simulink library "TripedLibraryMAX"  (R2021a)
settings: Solver=VariableStepAuto  StartTime=0.0  StopTime=10.0  RelTol=1e-3
undefined symbols (not defined in the file or in its folder): X, Y, Z
libraries: sm_lib x100, nesl_utility x31, $bdroot x27, robotlib x27, simulink x6
(part 5 of 6)

== system TripedLibraryMAX/Full Model/ROS/Subscribers/Subscriber_leg1/Ros-Subscriber-leg-1-left-swing-motor ==
  Bus Selector : BusSelector  OutputSignals=Position
  Constant : Constant  Value=[1]
  Selector : Selector  IndexOptions=Index vector (port)  Indices=[2]  OutputSizes=1
  Subscribe : robotlib/Subscribe  topicSource=Specify your own  topic=/leg_1/swing/left/joint/position_controller/command  messageType=sensor_msgs/JointState  SampleTime=-1  legacyBusStruct=off  messageQueueLength=1
  Terminator1 : Terminator
  swing_left_motor_position_OUT : Outport
  wires:
  Subscribe/1 -> Terminator1/1
  Subscribe/2 -> Bus Selector/1
  Constant/1 -> Selector/2
  Bus Selector/1 -> Selector/1  "<Position>"
  Selector/1 -> swing_left_motor_position_OUT/1

== system TripedLibraryMAX/Full Model/ROS/Subscribers/Subscriber_leg1/Ros-Subscriber-leg-1-right-swing-motor ==
  Bus Selector : BusSelector  OutputSignals=Position
  Constant : Constant  Value=[1]
  Selector : Selector  IndexOptions=Index vector (port)  Indices=[2]  OutputSizes=1
  Subscribe : robotlib/Subscribe  topicSource=Specify your own  topic=/leg_1/swing/right/joint/position_controller/command  messageType=sensor_msgs/JointState  SampleTime=-1  legacyBusStruct=off  messageQueueLength=1
  Terminator1 : Terminator
  swing_right_motor_position_OUT : Outport
  wires:
  Subscribe/1 -> Terminator1/1
  Subscribe/2 -> Bus Selector/1
  Constant/1 -> Selector/2
  Bus Selector/1 -> Selector/1  "<Position>"
  Selector/1 -> swing_right_motor_position_OUT/1

== system TripedLibraryMAX/Full Model/ROS/Subscribers/Subscriber_leg2/Ros-Subscriber-leg-2-extend-motor ==
  Bus Selector : BusSelector  OutputSignals=Position
  Constant : Constant  Value=[1]
  Selector : Selector  IndexOptions=Index vector (port)  Indices=[2]  OutputSizes=1
  Subscribe : robotlib/Subscribe  topicSource=Specify your own  topic=/leg_2/extend/joint/position_controller/command  messageType=sensor_msgs/JointState  SampleTime=-1  legacyBusStruct=off  messageQueueLength=1
  Terminator1 : Terminator
  extend_motor_position_OUT : Outport
  wires:
  Subscribe/1 -> Terminator1/1
  Subscribe/2 -> Bus Selector/1
  Bus Selector/1 -> Selector/1  "<Position>"
  Constant/1 -> Selector/2
  Selector/1 -> extend_motor_position_OUT/1

== system TripedLibraryMAX/Full Model/ROS/Subscribers/Subscriber_leg2/Ros-Subscriber-leg-2-left-swing-motor ==
  Bus Selector : BusSelector  OutputSignals=Position
  Constant : Constant  Value=[1]
  Selector : Selector  IndexOptions=Index vector (port)  Indices=[2]  OutputSizes=1
  Subscribe : robotlib/Subscribe  topicSource=Specify your own  topic=/leg_2/swing/left/joint/position_controller/command  messageType=sensor_msgs/JointState  SampleTime=-1  legacyBusStruct=off  messageQueueLength=1
  Terminator1 : Terminator
  swing_left_motor_position_OUT : Outport
  wires:
  Subscribe/1 -> Terminator1/1
  Subscribe/2 -> Bus Selector/1
  Constant/1 -> Selector/2
  Bus Selector/1 -> Selector/1  "<Position>"
  Selector/1 -> swing_left_motor_position_OUT/1

== system TripedLibraryMAX/Full Model/ROS/Subscribers/Subscriber_leg2/Ros-Subscriber-leg-2-right-swing-motor ==
  Bus Selector : BusSelector  OutputSignals=Position
  Constant : Constant  Value=[1]
  Selector : Selector  IndexOptions=Index vector (port)  Indices=[2]  OutputSizes=1
  Subscribe : robotlib/Subscribe  topicSource=Specify your own  topic=/leg_2/swing/right/joint/position_controller/command  messageType=sensor_msgs/JointState  SampleTime=-1  legacyBusStruct=off  messageQueueLength=1
  Terminator1 : Terminator
  swing_right_motor_position_OUT : Outport
  wires:
  Subscribe/1 -> Terminator1/1
  Subscribe/2 -> Bus Selector/1
  Constant/1 -> Selector/2
  Bus Selector/1 -> Selector/1  "<Position>"
  Selector/1 -> swing_right_motor_position_OUT/1

== matlab function TripedLibraryMAX/Full Model/ROS/Publishers/Publisher_leg_0/leg_0_extend_joint_pub/mergeValuesIntoRosMsg_test ==
  data: blankMessage input; position input; msg output Bus: SL_Bus_rosTest_sensor_msgs_JointState
function msg = assignNestedArrayMessages(blankMessage,position)
%#codegen

% To set datatype of MSG output:
%  1. Create buses for this model: robotics.ros.createSimulinkBus(gcs)
%  1. Click "Edit Data" in  Toolstrip to open "Ports and Data Manager"
%  2. Select MSG and set datatype to "Bus: SL_Bus_<modelname>_<messageType>"
% See: 
% http://www.mathworks.com/help/simulink/ug/create-structures-in-matlab-function-blocks.html

% blankMessage (geometry_msgs/PoseArray) consists of
%          Header: [1x1 Header]
%           Poses: variable-length array of geometry_msgs/Pose
%   Poses_SL_Info: struct with fields CurrentLength, ReceivedLength
%
% Poses is an array of "geometry_msgs/Pose", which consists of
%        Position: [1x1 Point]
%     Orientation: [1x1 Quaternion]
%
% Position is "geometry_msgs/Point", which consists of
%               X: 0
%               Y: 0
%               Z: 0
%
% Orientation is "geometry_msgs/Quaternion", which consists of
%               X: 0
%               Y: 0
%               Z: 0
%               W: 0

% Set the Poses array to have size 2, and modify the first two Pose
% elements

%blankMessage.Poses_SL_Info.CurrentLength = uint32(2);
%blankMessage.Poses(1).Position.X = x;
%blankMessage.Poses(1).Position.Y = y;
%blankMessage.Poses(2).Orientation.Z = z;

blankMessage.Position_SL_Info.CurrentLength = uint32(1);
%blankMessage.Velocity_SL_Info.CurrentLength = uint32(2);
%blankMessage.Effort_SL_Info.CurrentLength = uint32(2);
blankMessage.Position(1) = position;
%blankMessage.Velocity(2) = velocity;
%blankMessage.Position(2) = effort;

msg = blankMessage;

== matlab function TripedLibraryMAX/Examples/Full Body orientation and height control/fullbody kinematics ==
  data: foot0 input; res output; foot1 input; foot2 input; roll input; pitch input; yaw input; height input
function res  = fcn(foot0,foot1,foot2,roll,pitch,yaw,height)
floor_ccs_trafo=transform_to_floor(roll,pitch,yaw,height);

ccs_foot0=floor_ccs_trafo*makehomogenous(foot0);
ccs_foot1=floor_ccs_trafo*makehomogenous(foot1);
ccs_foot2=floor_ccs_trafo*makehomogenous(foot2);
res=[ccs_foot0(1:3);ccs_foot1(1:3);ccs_foot2(1:3)];
end


function [transfM] = transform_to_floor(roll,pitch,yaw,height)
%first translate frame by [x,y,z]
transfM1 = homogenTranslationMat(0,0,-height);
%then rotate using the roll-pitch-yaw convention
transfM2 = makeRotHomogen(eul2rotm([-roll,-pitch,-yaw],'XYZ'));
transfM = transfM1 * transfM2;
end

function [res] = makehomogenous(vector)
    res = [vector(1);vector(2);vector(3);1];
end

function [res] = homogenTranslationMat(x,y,z)
    %this function forms a Translation about a vector x,y,z in a homogenous
    %transformation matrix res. 
    res = [1 0 0 x; 0 1 0 y; 0 0 1 z; 0 0 0 1];
end

function [res] = makeRotHomogen(R)
% this function fills a Rotationmatrix to make it a homogen transformation
% matrix
res = [R(1,1) R(1,2) R(1,3) 0; R(2,1) R(2,2) R(2,3) 0; R(3,1) R(3,2) R(3,3) 0; 0 0 0 1];
end

== matlab function TripedLibraryMAX/Full Model/ROS/Publishers/Publisher_leg_1/leg_1_swing_right_joint_pub/mergeValuesIntoRosMsg_test ==
  data: blankMessage input; position input; msg output Bus: SL_Bus_rosTest_sensor_msgs_JointState
function msg = assignNestedArrayMessages(blankMessage,position)
%#codegen

% To set datatype of MSG output:
%  1. Create buses for this model: robotics.ros.createSimulinkBus(gcs)
%  1. Click "Edit Data" in  Toolstrip to open "Ports and Data Manager"
%  2. Select MSG and set datatype to "Bus: SL_Bus_<modelname>_<messageType>"
% See: 
% http://www.mathworks.com/help/simulink/ug/create-structures-in-matlab-function-blocks.html

% blankMessage (geometry_msgs/PoseArray) consists of
%          Header: [1x1 Header]
%           Poses: variable-length array of geometry_msgs/Pose
%   Poses_SL_Info: struct with fields CurrentLength, ReceivedLength
%
% Poses is an array of "geometry_msgs/Pose", which consists of
%        Position: [1x1 Point]
%     Orientation: [1x1 Quaternion]
%
% Position is "geometry_msgs/Point", which consists of
%               X: 0
%               Y: 0
%               Z: 0
%
% Orientation is "geometry_msgs/Quaternion", which consists of
%               X: 0
%               Y: 0
%               Z: 0
%               W: 0

% Set the Poses array to have size 2, and modify the first two Pose
% elements

%blankMessage.Poses_SL_Info.CurrentLength = uint32(2);
%blankMessage.Poses(1).Position.X = x;
%blankMessage.Poses(1).Position.Y = y;
%blankMessage.Poses(2).Orientation.Z = z;

blankMessage.Position_SL_Info.CurrentLength = uint32(1);
%blankMessage.Velocity_SL_Info.CurrentLength = uint32(2);
%blankMessage.Effort_SL_Info.CurrentLength = uint32(2);
blankMessage.Position(1) = position;
%blankMessage.Velocity(2) = velocity;
%blankMessage.Position(2) = effort;

msg = blankMessage;

== matlab function TripedLibraryMAX/Examples/rabbid walking/fullbody kinematics ==
  data: foot0 input; res output; foot1 input; foot2 input; roll input; pitch input; yaw input; height input
function res  = fcn(foot0,foot1,foot2,roll,pitch,yaw,height)
floor_ccs_trafo=transform_to_floor(roll,pitch,yaw,height);

ccs_foot0=floor_ccs_trafo*makehomogenous(foot0);
ccs_foot1=floor_ccs_trafo*makehomogenous(foot1);
ccs_foot2=floor_ccs_trafo*makehomogenous(foot2);
res=[ccs_foot0(1:3);ccs_foot1(1:3);ccs_foot2(1:3)];
end


function [transfM] = transform_to_floor(roll,pitch,yaw,height)
%first translate frame by [x,y,z]
transfM1 = homogenTranslationMat(0,0,-height);
%then rotate using the roll-pitch-yaw convention
transfM2 = makeRotHomogen(eul2rotm([-roll,-pitch,-yaw],'XYZ'));
transfM = transfM1 * transfM2;
end

function [res] = makehomogenous(vector)
    res = [vector(1);vector(2);vector(3);1];
end

function [res] = homogenTranslationMat(x,y,z)
    %this function forms a Translation about a vector x,y,z in a homogenous
    %transformation matrix res. 
    res = [1 0 0 x; 0 1 0 y; 0 0 1 z; 0 0 0 1];
end

function [res] = makeRotHomogen(R)
% this function fills a Rotationmatrix to make it a homogen transformation
% matrix
res = [R(1,1) R(1,2) R(1,3) 0; R(2,1) R(2,2) R(2,3) 0; R(3,1) R(3,2) R(3,3) 0; 0 0 0 1];
end

== matlab function TripedLibraryMAX/Kinematic Utils/Simplified  Model Kinematics/Fwd Kin ==
  data: Jointstate input; leg_pos output; LegNumber input
function leg_pos = fcn(Jointstate, LegNumber)
waym = Weg0(eul2quat(Jointstate(1:3).','XYZ'));
wayLLm = AddLegLinear(waym,Jointstate(4));
legstate = getPosfromHomogenMatrix(wayLLm);
leg_pos = transformCoordinateSystem(legstate(1),legstate(2),legstate(3),LegNumber);
end




function [new_pos] = transformCoordinateSystem(oldx,oldy,oldz,legNr)
% this function translates the x,y,z coordinates from the CCS into
% coordinates from a cooridnate system, which is like the ccs but rotated
% ,such that its x axis points towards the leg. This makes the inverse
% kinematics for the leg 1 & 2 easier.

angle = -legNr * 120 * pi/180;

newCoord = [cos(angle), -sin(angle), 0, 0; sin(angle), cos(angle), 0, 0; 0, 0, 1, 0; 0, 0, 0, 1]* [oldx;oldy;oldz;1];
newx = newCoord(1);
newy = newCoord(2);
newz = newCoord(3);

new_pos=[newx;newy;newz];
end

function [Weg0Mat] = Weg0(quat)
a0=quat(1);
b0=quat(2);
c0=quat(3);
d0=quat(4);
A1 = homogenTranslationMat(0.265,0,0.014);
A2 = makeRotHomogen(quatRotM(a0,b0,c0,d0));
Weg0Mat = A1 * A2;
end



function [ALeg] = AddLegLinear(A,theta0)
% this functions adds transformations from the Point P; which is the
% Endpoint from Weg0,1,2; to the FKS at the LegLinear part.
%{
Inputs:
A: Transformationmatrix, which describes Transformation from HKS to P, Use
Weg0.m for that.
theta0: angle [radians] for joint at MKS00
%}

A1 = homogenTranslationMat(1.640, 0, -0.037); % transform P to P0 (center of LegLinear rotation)

theta0 = -1* theta0 * 0.07/(2*pi*1.5); % gear constraint: transfrom theta at MKS00 to theta around P0, gear ratio is 0.07/(2*pi*1.5) [circumferences of the gears MCS00 / LL]
startingvalue = -0 * pi/180;
theta0 = theta0 + startingvalue; %offset, depending on which position the Leg is for theta at MKS00 is 0
A2 = makeRotHomogen(quatRotM(cos(theta0/2),0,sin(theta0/2),0)); % rotate theta [radians] around y-axis of P0

A3 = homogenTranslationMat(-1.500,0,0); %transform from P0-joint to FKS

ALeg = A*A1*A2*A3;
end

function [res] = homogenTranslationMat(x,y,z)
    %this function forms a Translation about a vector x,y,z in a homogenous
    %transformation matrix res. 
    res = [1 0 0 x; 0 1 0 y; 0 0 1 z; 0 0 0 1];
end

function [res] = makeRotHomogen(R)
% this function fills a Rotationmatrix to make it a homogen transformation
% matrix
res = [R(1,1) R(1,2) R(1,3) 0; R(2,1) R(2,2) R(2,3) 0; R(3,1) R(3,2) R(3,3) 0; 0 0 0 1];
end

function [res] = quatRotM(a,b,c,d) %%Todo: use a,b,c,d as inputs
    % this function calculates the quaternion rotation matrix for given
    % vector (kx,ky,kz) and angle theta
    res = [1-2*(c^2+d^2) 2*(b*c-d*a) 2*(b*d + c*a); 2*(b*c + d*a) 1-2*(b^2+d^2) 2*(c*d - b*a); 2*(b*d-c*a) 2*(c*d+b*a) 1-2*(b^2+c^2) ];
end




function [pos] = getPosfromHomogenMatrix(A)
    % this function returns the Vector by which a homogenous
    % Transformationmatrix shifts a Point
    x = A(1,4);
    y = A(2,4);
    z = A(3,4);

    pos=[x,y,z];
end

== matlab function TripedLibraryMAX/Kinematic Utils/Simplified  Model Kinematics/Inv Kin/Simplified_inverse_kinematics ==
  data: extend output; Rx output; Ry output; x input; y input; z input; LegAngle input; Rz output; data input; Residual output
function [extend,Rx,Ry,Rz,Residual]= fcn(x,y,z,LegAngle, data)



% easy adapt to legs 1 & 2: change base coordinate system from ccs, to
% rotated ccs.
[newx,newy,newz] = transformCoordinateSystem(x,y,z,LegAngle);

% calculate target Matrix from input cordinates
%reduced task space to x,y,z, ignore roll, pitch, yaw
[targetM] = convertCoordinatesToMatrix(newx,newy,newz);

%convert euer angles from data to quaternion
guess = convertDataToGuess(data);

[extend, a0,b0,c0,d0, Residual] = inverseKinematics(targetM,guess);
eulerXYZ = quat2eul([a0,b0,c0,d0],'XYZ');
Rx = eulerXYZ(1);
Ry = eulerXYZ(2);
Rz = eulerXYZ(3);

end

function [newx,newy,newz] = transformCoordinateSystem(oldx,oldy,oldz,legAngle)
% this function translates the x,y,z coordinates from the CCS into
% coordinates from a cooridnate system, which is like the ccs but rotated
% ,such that its x axis points towards the leg. This makes the inverse
% kinematics for the leg 1 & 2 easier.

angle = legAngle;

newCoord = [cos(angle), -sin(angle), 0, 0; sin(angle), cos(angle), 0, 0; 0, 0, 1, 0; 0, 0, 0, 1]* [oldx;oldy;oldz;1];
newx = newCoord(1);
newy = newCoord(2);
newz = newCoord(3);

end

function [transfM] = convertCoordinatesToMatrix(x,y,z)
transfM = homogenTranslationMat(x,y,z);
end

function [guess] = convertDataToGuess(data)
    qXYZ = eul2quat([data(1),data(2),data(3)],'XYZ');
    guess = [qXYZ,data(4)];    
end

function [theta0,a0,b0,c0,d0,fvalWay0] = inverseKinematics(A,guess)
% maps a homogene transformation matrix, which describes the desired position
% of the fcs relative to the ccs in taskspace to the joint space variables


options = optimoptions(@fmincon,'OptimalityTolerance',10^-10,'StepTolerance',10^-10,'display','off','Algorithm','sqp'); % some options for more precise solution


guessVals = guess;

clW0toTarget = @(guessVals)closingWay0toTarget(guessVals,A);
[OptVals,fvalWay0,~,~] = fmincon(clW0toTarget,guessVals,[],[],[],[],[],[],@constrainsWay0,options);

% get values from way0
a0 = OptVals(1);
b0 = OptVals(2);
c0 = OptVals(3);
d0 = OptVals(4);
theta0 = OptVals(5);

end


function [error] = closingWay0toTarget(GUESSEDVALS,MatA)
% this function returns the error between the desired fcs position and the
% fcs position given GUESSEDVALS as joint values

a0 = GUESSEDVALS(1);
b0 = GUESSEDVALS(2);
c0 = GUESSEDVALS(3);
d0 = GUESSEDVALS(4);
theta0 = GUESSEDVALS(5);

way0m = Weg0(a0,b0,c0,d0);
way0LLm = AddLegLinear(way0m,theta0);
[a,b,c,d, x,y,z] = getValuesfromHomogenMatrix(way0LLm);
[~,~,~,~, Ax,Ay,Az] = getValuesfromHomogenMatrix(MatA);


% give the system a desired yaw of the leg, since it is highly redundant
% this makes the solution more predictable

[~,desired_orientation]= cart2pol(Ax-0.265,Ay);
current_orientation = quat2eul([a0,b0,c0,d0]);


error = sum(([x,y,z] - [Ax,Ay,Az]).^2) + 1*(a^2+b^2+c^2+d^2 -1)^2+10*(desired_orientation-current_orientation(1))^2; % error of the transform to A. we want to minimze this
end


function [c,ceq]= constrainsWay0(x)
% make sure that solver fmincon considers that the quaternion a0,b0,c0,d0
% needs to be normalized.
%input: x = [a0,b0,c0,d0,theta0].
c = []; % condition for ... <= 0
ceq = x(1)^2+x(2)^2+x(3)^2+x(4)^2 - 1; % condition for ||quat0|| == 1    
end

function [Weg0Mat] = Weg0(a0,b0,c0,d0)
A1 = homogenTranslationMat(0.265,0,0.014);
A2 = makeRotHomogen(quatRotM(a0,b0,c0,d0));
Weg0Mat = A1 * A2;
end

function [ALeg] = AddLegLinear(A,theta0)
% this functions adds transformations from the Point P; which is the
% Endpoint from Weg0,1,2; to the FKS at the LegLinear part.
%{
Inputs:
A: Transformationmatrix, which describes Transformation from HKS to P, Use
Weg0.m for that.
theta0: angle [radians] for joint at MKS00
%}

A1 = homogenTranslationMat(1.640, 0, -0.037); 

theta0 = -1* theta0 * 0.07/(2*pi*1.5); % gear constraint between motor and curved leg
startingvalue = -0 * pi/180;
theta0 = theta0 + startingvalue;
A2 = makeRotHomogen(quatRotM(cos(theta0/2),0,sin(theta0/2),0)); 
A3 = homogenTranslationMat(-1.500,0,0);

ALeg = A*A1*A2*A3;
end

function [res] = homogenTranslationMat(x,y,z)
    %this function forms a Translation about a vector x,y,z in a homogenous
    %transformation matrix res. 
    res = [1 0 0 x; 0 1 0 y; 0 0 1 z; 0 0 0 1];
end

function [res] = makeRotHomogen(R)
% this function fills a Rotationmatrix to make it a homogen transformation
% matrix
res = [R(1,1) R(1,2) R(1,3) 0; R(2,1) R(2,2) R(2,3) 0; R(3,1) R(3,2) R(3,3) 0; 0 0 0 1];
end

function [res] = quatRotM(a,b,c,d) %%Todo: use a,b,c,d as inputs
    res = [1-2*(c^2+d^2) 2*(b*c-d*a) 2*(b*d + c*a); 2*(b*c + d*a) 1-2*(b^2+d^2) 2*(c*d - b*a); 2*(b*d-c*a) 2*(c*d+b*a) 1-2*(b^2+c^2) ];
end

function [a,b,c,d, x,y,z] = getValuesfromHomogenMatrix(A)
    % this function returns the Vector by which a homogenous
    % Transformationmatrix shifts a Point
    x = A(1,4);
    y = A(2,4);
    z = A(3,4);

    %as well as the Values of the Quaternion describing the rotation
    R = A(1:3,1:3); % get Rot mat from homogen Mat
    quat = rotm2quat(R);
    a = quat(1);
    b = quat(2);
    c = quat(3);
    d = quat(4); 
end

== matlab function TripedLibraryMAX/Full Model/ROS/Publishers/Publisher_leg_2/leg_2_extend_joint_pub/mergeValuesIntoRosMsg_test ==
  data: blankMessage input; position input; msg output Bus: SL_Bus_rosTest_sensor_msgs_JointState
function msg = assignNestedArrayMessages(blankMessage,position)
%#codegen

% To set datatype of MSG output:
%  1. Create buses for this model: robotics.ros.createSimulinkBus(gcs)
%  1. Click "Edit Data" in  Toolstrip to open "Ports and Data Manager"
%  2. Select MSG and set datatype to "Bus: SL_Bus_<modelname>_<messageType>"
% See: 
% http://www.mathworks.com/help/simulink/ug/create-structures-in-matlab-function-blocks.html

% blankMessage (geometry_msgs/PoseArray) consists of
%          Header: [1x1 Header]
%           Poses: variable-length array of geometry_msgs/Pose
%   Poses_SL_Info: struct with fields CurrentLength, ReceivedLength
%
% Poses is an array of "geometry_msgs/Pose", which consists of
%        Position: [1x1 Point]
%     Orientation: [1x1 Quaternion]
%
% Position is "geometry_msgs/Point", which consists of
%               X: 0
%               Y: 0
%               Z: 0
%
% Orientation is "geometry_msgs/Quaternion", which consists of
%               X: 0
%               Y: 0
%               Z: 0
%               W: 0

% Set the Poses array to have size 2, and modify the first two Pose
% elements

%blankMessage.Poses_SL_Info.CurrentLength = uint32(2);
%blankMessage.Poses(1).Position.X = x;
%blankMessage.Poses(1).Position.Y = y;
%blankMessage.Poses(2).Orientation.Z = z;

blankMessage.Position_SL_Info.CurrentLength = uint32(1);
%blankMessage.Velocity_SL_Info.CurrentLength = uint32(2);
%blankMessage.Effort_SL_Info.CurrentLength = uint32(2);
blankMessage.Position(1) = position;
%blankMessage.Velocity(2) = velocity;
%blankMessage.Position(2) = effort;

msg = blankMessage;

== matlab function TripedLibraryMAX/Full Model/ROS/Publishers/Publisher_leg_2/leg_2_swing_left_joint_pub/mergeValuesIntoRosMsg_test ==
  data: blankMessage input; position input; msg output Bus: SL_Bus_rosTest_sensor_msgs_JointState
function msg = assignNestedArrayMessages(blankMessage,position)
%#codegen

% To set datatype of MSG output:
%  1. Create buses for this model: robotics.ros.createSimulinkBus(gcs)
%  1. Click "Edit Data" in  Toolstrip to open "Ports and Data Manager"
%  2. Select MSG and set datatype to "Bus: SL_Bus_<modelname>_<messageType>"
% See: 
% http://www.mathworks.com/help/simulink/ug/create-structures-in-matlab-function-blocks.html

% blankMessage (geometry_msgs/PoseArray) consists of
%          Header: [1x1 Header]
%           Poses: variable-length array of geometry_msgs/Pose
%   Poses_SL_Info: struct with fields CurrentLength, ReceivedLength
%
% Poses is an array of "geometry_msgs/Pose", which consists of
%        Position: [1x1 Point]
%     Orientation: [1x1 Quaternion]
%
% Position is "geometry_msgs/Point", which consists of
%               X: 0
%               Y: 0
%               Z: 0
%
% Orientation is "geometry_msgs/Quaternion", which consists of
%               X: 0
%               Y: 0
%               Z: 0
%               W: 0

% Set the Poses array to have size 2, and modify the first two Pose
% elements

%blankMessage.Poses_SL_Info.CurrentLength = uint32(2);
%blankMessage.Poses(1).Position.X = x;
%blankMessage.Poses(1).Position.Y = y;
%blankMessage.Poses(2).Orientation.Z = z;

blankMessage.Position_SL_Info.CurrentLength = uint32(1);
%blankMessage.Velocity_SL_Info.CurrentLength = uint32(2);
%blankMessage.Effort_SL_Info.CurrentLength = uint32(2);
blankMessage.Position(1) = position;
%blankMessage.Velocity(2) = velocity;
%blankMessage.Position(2) = effort;

msg = blankMessage;

== matlab function TripedLibraryMAX/Full Model/ROS/Publishers/Publisher_leg_2/leg_2_swing_right_joint_pub/mergeValuesIntoRosMsg_test ==
  data: blankMessage input; position input; msg output Bus: SL_Bus_rosTest_sensor_msgs_JointState
function msg = assignNestedArrayMessages(blankMessage,position)
%#codegen

% To set datatype of MSG output:
%  1. Create buses for this model: robotics.ros.createSimulinkBus(gcs)
%  1. Click "Edit Data" in  Toolstrip to open "Ports and Data Manager"
%  2. Select MSG and set datatype to "Bus: SL_Bus_<modelname>_<messageType>"
% See: 
% http://www.mathworks.com/help/simulink/ug/create-structures-in-matlab-function-blocks.html

% blankMessage (geometry_msgs/PoseArray) consists of
%          Header: [1x1 Header]
%           Poses: variable-length array of geometry_msgs/Pose
%   Poses_SL_Info: struct with fields CurrentLength, ReceivedLength
%
% Poses is an array of "geometry_msgs/Pose", which consists of
%        Position: [1x1 Point]
%     Orientation: [1x1 Quaternion]
%
% Position is "geometry_msgs/Point", which consists of
%               X: 0
%               Y: 0
%               Z: 0
%
% Orientation is "geometry_msgs/Quaternion", which consists of
%               X: 0
%               Y: 0
%               Z: 0
%               W: 0

% Set the Poses array to have size 2, and modify the first two Pose
% elements

%blankMessage.Poses_SL_Info.CurrentLength = uint32(2);
%blankMessage.Poses(1).Position.X = x;
%blankMessage.Poses(1).Position.Y = y;
%blankMessage.Poses(2).Orientation.Z = z;

blankMessage.Position_SL_Info.CurrentLength = uint32(1);
%blankMessage.Velocity_SL_Info.CurrentLength = uint32(2);
%blankMessage.Effort_SL_Info.CurrentLength = uint32(2);
blankMessage.Position(1) = position;
%blankMessage.Velocity(2) = velocity;
%blankMessage.Position(2) = effort;

msg = blankMessage;

== matlab function TripedLibraryMAX/Kinematic Utils/Full Model kinematics/Full_Fwd_Kin ==
  data: Jointstate input; leg_pos output; LegNumber input; residual output
function [leg_pos, residual]  = forwardKinematics(Jointstate, LegNumber)
% Jointstate = [sw_left,extend, sw_right] each as an angle to rotate about
% their z
% LegNumber = Identifier of current Leg. [0-2]
% data: states from previous iteration
% leg_pos = [x,y,z] position of the fcs relative to the ccs of that leg 

%Todo: add previos step as initial guess
 
%% solve closed kinematic chain

options = optimoptions(@fmincon,'OptimalityTolerance',10^-10,'StepTolerance',10^-10,'display','off','Algorithm','sqp'); % some options for more precise solution

%[LCS,left_sph1,left_sph2,right_sph1,right_sph2]
guessedVals = [0.9961, -0.0000, -0.0893, 0.0000, 0.8135, 0.0244, -0.0019, -0.5821, 0.9338, 0.0064, -0.0889, 0.3413, 0.8135, -0.0245, -0.0019, 0.5821, -0.9338, 0.0064, 0.0889, 0.3413];


clE = @(guessedVals)closingEquation(guessedVals,Jointstate(1),Jointstate(3)); % LCS, sphs; sw_left, sw_right
constraints = @(guessedVals)constrainsClosedChain(guessedVals);
[OptVals,residual,~,~] = fmincon(clE,guessedVals,[],[],[],[],[],[],constraints,options);

A_closed = bridgeSubChainM(OptVals(1:4)); % calculate P frame from optimized joinststate

%% Add open subchain

A_open = openSubChain(Jointstate(2));
A_leg = A_closed * A_open;

%% rotate to transform into correct Leg
leg_pos = transformCoordinateSystem(A_leg(1,4),A_leg(2,4),A_leg(3,4),LegNumber);

end
%% Helper functions

%% Helper - setup transformations
function [res] = homogenTranslationMat(x,y,z)
    %this function forms a Translation about a vector x,y,z in a homogenous
    %transformation matrix res. 
    res = [1 0 0 x; 0 1 0 y; 0 0 1 z; 0 0 0 1];
end

function [res] = makeRotHomogen(R)
% this function fills a Rotationmatrix to make it a homogen transformation
% matrix
res = [R(1,1) R(1,2) R(1,3) 0; R(2,1) R(2,2) R(2,3) 0; R(3,1) R(3,2) R(3,3) 0; 0 0 0 1];
end

function [res] = quatRotM(a,b,c,d) %%Todo: use a,b,c,d as inputs
    % this function calculates the quaternion rotation matrix for given
    % vector (kx,ky,kz) and angle theta
    res = [1-2*(c^2+d^2) 2*(b*c-d*a) 2*(b*d + c*a); 2*(b*c + d*a) 1-2*(b^2+d^2) 2*(c*d - b*a); 2*(b*d-c*a) 2*(c*d+b*a) 1-2*(b^2+c^2) ];
end

function [res] = homoQuatTransfM(quat,x,y,z)
    % homogen Transformation matrix using quaternion and xyz vector
    res = [quatRotM(quat(1),quat(2),quat(3),quat(4)),[x;y;z];[0,0,0,1]];
end

function [res] = homoRotZTransfM(angle,x,y,z)
    % homogen Transformation matrix using rotation around z and xyz vector
    res = [[cos(angle), -sin(angle), 0; sin(angle), cos(angle), 0; 0,0,1],[x;y;z];[0,0,0,1]];
end


function [res] = homoRotZM(a)
% homogen tranfs mat describing a z rotation about a radians
    res = [cos(a), -sin(a), 0,0; sin(a), cos(a), 0,0; 0,0,1,0; 0,0,0,1];
end

function [a,b,c,d, x,y,z] = getValuesfromHomogenMatrix(A)
    % this function returns the Vector by which a homogenous
    % Transformationmatrix shifts a Point
    x = A(1,4);
    y = A(2,4);
    z = A(3,4);

    %as well as the Values of the Quaternion describing the rotation
    R = A(1:3,1:3); % get Rot mat from homogen Mat
    quati = rotm2quat(R); % convert to quaternion
    quati = quaternion(quati);
    [a,b,c,d] = parts(quati); % get parts from quaternion  
    
end


%% Subchains

function [res] = bridgeSubChainM(lcsQuat)
 % transformation matrix for middle sub sub chain (LCS) of closed sub chain
    a0=lcsQuat(1);
    b0=lcsQuat(2);
    c0=lcsQuat(3);
    d0=lcsQuat(4);
    A1 = homogenTranslationMat(0.265,0,0.014);
    A2 = makeRotHomogen(quatRotM(a0,b0,c0,d0));
    res = A1 * A2;
end


function [res] = leftSubChain(leftSwAngle,spJoint1Quat, spJoint2Quat)
% transformation matrix of left sub sub chain (left swing joint) of closed sub chain
% inputs: 
%   leftSwAngle: double, Angle of the left swing joint
%   spJoint1Quat: quaternion (4x double), quaternion for 1st spherical
%   Joint in coupling rod
%   spJoint2Quat: quaternion (4x double), quaternion for 2nd spherical
%   Joint in coupling rod

angle_ccs_to_leftSw = -21.4745 * pi / 180; % the angle between the x-Axes CCS --> leftSW [theta = 0]

A_ccs_leftSw = homoRotZTransfM(angle_ccs_to_leftSw, 0.139807669447128, -0.0549998406976098, -0.051); % CCS --> left swing base
A_leftSW = homoRotZM(leftSwAngle); % left swing joint base --> follower
A_leftSW_sph1 = homoQuatTransfM(spJoint1Quat,0.085,0,-0.0245); % follower to spherical joint 1 follower of coupling rod
A_sph1_sph2 = homoQuatTransfM(spJoint2Quat,0.11,0,0); % sph1 follower to sph2 follower of coupling rod
A_sph2_P = homogenTranslationMat(-0.015,0.0299,0.0965); % spherical joint 2 to endpoint P of closed chain

res = A_ccs_leftSw * A_leftSW * A_leftSW_sph1 * A_sph1_sph2 * A_sph2_P; % chain transformations

end

function [res] = rightSubChain(rightSwAngle,spJoint1Quat, spJoint2Quat)
% transformation matrix of right sub sub chain (right swing joint) of closed sub chain
% inputs: 
%   rightSwAngle: double, Angle of the right swing joint
%   spJoint1Quat: quaternion (4x double), quaternion for 1st spherical
%   Joint in coupling rod
%   spJoint2Quat: quaternion (4x double), quaternion for 2nd spherical
%   Joint in coupling rod

angle_ccs_to_rightSw = -338.5255 * pi / 180; % the angle between the x-Axes CCS --> rightSW [theta = 0]

A_ccs_rightSw = homoRotZTransfM(angle_ccs_to_rightSw, 0.139807669447128, 0.0549998406976098, -0.051); % CCS --> right swing base
A_rightSW = homoRotZM(rightSwAngle); % right swing joint base --> follower
A_rightSW_sph1 = homoQuatTransfM(spJoint1Quat,0.085,0,-0.0245); % follower to spherical joint 1 follower of coupling rod
A_sph1_sph2 = homoQuatTransfM(spJoint2Quat,0.11,0,0); % sph1 follower to sph2 follower of coupling rod
A_sph2_P = homogenTranslationMat(-0.015,-0.0299,0.0965); % spherical joint 2 to endpoint P of closed chain

res = A_ccs_rightSw * A_rightSW * A_rightSW_sph1 * A_sph1_sph2 * A_sph2_P; % chain transformations

end

function [res] = openSubChain(theta0)
% From endpoint of closed sub chain to fcs
%{
Inputs:
angle [radians] for extendJoint (Motor)
%}

A_P_llJointBase = homogenTranslationMat(1.640, 0, -0.037); % transform P to P0 (center of LegLinear rotation)

startingValue = -3 * pi/180; % Zero-Offset for ExtendJoint
% gear constraint: transfrom theta at MKS00 to theta around P0, gear ratio is 0.07/(2*pi*1.5) [circumferences of the gears MCS00 / LL]
%theta0 = -1* angle * 0.07/(2*pi*1.5); 
theta0 = theta0 + startingValue; %offset, depending on which position the Leg is for theta at MKS00 is 0

% LL-Joint base --> follower
A_llJoint = makeRotHomogen(quatRotM(cos(theta0/2),0,sin(theta0/2),0)); % rotate theta [radians] around y-axis of LL_joint base

A_follower_fcs = homogenTranslationMat(-1.500,0,0); %transform from LL-joint to FKS

res = A_P_llJointBase*A_llJoint*A_follower_fcs;
end

%% closing equations

function [totalError] = closingEquation(GUESSEDVALS,theta1,theta2) % for use in fminunc

%GUESSEDVALS = num2cell(GUESSEDVALS);
%[a0,b0,c0,d0,a1_1,b1_1,c1_1,d1_1, a1_2,b1_2,c1_2,d1_2,a2_1,b2_1,c2_1,d2_1, a2_2,b2_2,c2_2,d2_2] = GUESSEDVALS{:};

% set const values for theta. These can be taken from the simulation, but
% not given to closingEq() as variables, do to fminunc changing them


% this function returns the value of the closing equation for this Robot
%Input: TransformMatrices A,B,C of the 3 paths
A = bridgeSubChainM(GUESSEDVALS(1:4));
B = leftSubChain(theta1, GUESSEDVALS(5:8),GUESSEDVALS(9:12));
C = rightSubChain(theta2,GUESSEDVALS(13:16), GUESSEDVALS(17:20));


%equation = [A-B;B-C;C-A] == zeros(3*4,4); %% closure Equation. this is a LGS with 12 equations

% get relevant Values from the Matrices
[Aa,Ab,Ac,Ad, Ax,Ay,Az] = getValuesfromHomogenMatrix(A);
[Ba,Bb,Bc,Bd, Bx,By,Bz] = getValuesfromHomogenMatrix(B);
[Ca,Cb,Cc,Cd, Cx,Cy,Cz] = getValuesfromHomogenMatrix(C);

% evaluate closure Equation: ||A-B||^2 + ||A-C|| ^2 + ||B-C||^2
% we use the values from the matrices: (Ax-Bx)^2 + (Ay-By)^2 + (Az-Bz)^2
% + (Ax-Cx)^2 + ... + (Bx-Cx)^2 + ...
% as evaluation for the position error between the paths
Apos = [Ax;Ay;Az];
Bpos = [Bx;By;Bz];
Cpos = [Cx;Cy;Cz];
%PosError = sum((Apos-Bpos).^2 + (Apos-Cpos).^2 + (Bpos-Cpos).^2);
PosError = [Apos-Bpos;Bpos-Cpos;Cpos-Apos];

Arot = [Aa;Ab;Ac;Ad];
Brot = [Ba;Bb;Bc;Bd];
Crot = [Ca;Cb;Cc;Cd];

% for the rotational error we check the parts of the quaternions which
% describe the rotation for each path
% TODO: I'm not sure if they show errors where there are none though.
%RotError = sum((Arot-Brot).^2 + (Arot-Crot).^2 + (Brot-Crot).^2);
RotError = [Arot-Brot;Brot-Crot;Crot-Arot];

%totalError = PosError + RotError;
totalError = [PosError;RotError];
%totalError = norm(totalError)^2;
totalError = sum(totalError.^2);
end

%% constraints

function [c,ceq]= constrainsClosedChain(x)
% make sure that solver fmincon, solves with constraint : ||quati_j|| == 1
%input: x = [a0,...,d0,a1_1,...,d1_2,a2_1,...,d2_2].
c = []; % condition for ... <= 0
ceq0 = x(1)^2+x(2)^2+x(3)^2+x(4)^2 - 1; % condition for ||quat0|| == 1
ceq1_1 = x(5)^2+x(6)^2+x(7)^2+x(8)^2 - 1; % condition for ||quat1_1|| == 1
ceq1_2 = x(9)^2+x(10)^2+x(11)^2+x(12)^2 - 1; % condition for ||quat1_2|| == 1
ceq2_1 = x(13)^2+x(14)^2+x(15)^2+x(16)^2 - 1; % condition for ||quat2_1|| == 1
ceq2_2 = x(17)^2+x(18)^2+x(19)^2+x(20)^2 - 1; % condition for ||quat2_2|| == 1
ceq = [ceq0;ceq1_1;ceq1_2;ceq2_1;ceq2_2];
end

%% Helper - rotate into correct leg
function [new_pos] = transformCoordinateSystem(oldx,oldy,oldz,legNr)
% this function translates the x,y,z coordinates from the CCS into
% coordinates from a cooridnate system, which is like the ccs but rotated
% ,such that its x axis points towards the leg. This makes the inverse
% kinematics for the leg 1 & 2 easier.

angle = -legNr * 120 * pi/180;

newCoord = [cos(angle), -sin(angle), 0, 0; sin(angle), cos(angle), 0, 0; 0, 0, 1, 0; 0, 0, 0, 1]* [oldx;oldy;oldz;1];
newx = newCoord(1);
newy = newCoord(2);
newz = newCoord(3);

new_pos=[newx;newy;newz];
end

== matlab function TripedLibraryMAX/Kinematic Utils/Full Model kinematics/Full_Inv_Kin/Full_Inv_Kin_Fct ==
  data: legX input; swing_left output; legY input; legZ input; legNumber input; data input; extend output; swing_right output; newData output
function [swing_left,extend,swing_right, newData]= inverseKinematics(legX,legY,legZ,legNumber, data)
% calculates joint states for swing_left/right & extend joint for given
% traget fcs position
%inputs:
% legX,legY,legZ: double, position of fcs of specified leg, relative to ccs
% legNumber: int, specifies leg
% data: joint states of previous iteration used as initial state for solver
% [sw_left;extend;sw_right;LCS;left_sph1;left_sph2;right_sph1;right_sph2],
% with sw_left/right & extend as double; LCS & sph1/2 as quaternions

%outputs:
% swing_left,extend,swing_right: joint states for given target
% residual: solver error, useful for chekcing quality of solution
% newData: joint states of current iteration, feedback with delay for next iteration

% easy adapt to legs 1 & 2: change base coordinate system from ccs, to
% rotated ccs.
% rotate ccs around z s.t. x axis points in leg direction
% get new target position
[newx,newy,newz] = transformCoordinateSystem(legX,legY,legZ,legNumber);

% calculate target Matrix from input cordinates
%reduced task space to x,y,z, ignore roll, pitch, yaw
[targetM] = homogenTranslationMat(newx,newy,newz);

% solve inverse kinematics

%swing_left = 0.;
%swing_right = 0.;
%extend = 0.;
%residual = 0.;
%newData = zeros(23,1);

%coder.extrinsic('inverseKinematicsSolver'); % maybe this fixes the error: optimoptions not supported for code gen
[extend,swing_left,swing_right, newData] = inverseKinematicsSolver(targetM,data);

end

% initial data = [
% [-0.1745; 0;      0.1745 ; % sw left, extend, sw right
% 0.9961;   0.;     -0.0893; 0.; % LCS
% 0.8135;   0.0244; -0.0019; -0.5821; % left sph1
% 0.9338;   0.0064; -0.0889; 0.3413; % left sph2
% 0.8135; - 0.0245; -0.0019; 0.5821; % right sph1
% -0.9338;  0.0064; 0.0889;  0.3413] % right sph2
%]

function [newx,newy,newz] = transformCoordinateSystem(oldx,oldy,oldz,legNr)
% this function translates the x,y,z coordinates from the CCS into
% coordinates from a cooridnate system, which is like the ccs but rotated
% ,such that its x axis points towards the leg. This makes the inverse
% kinematics for the leg 1 & 2 easier.

angle = legNr * -120 * pi/180;

newCoord = [cos(angle), -sin(angle), 0, 0; sin(angle), cos(angle), 0, 0; 0, 0, 1, 0; 0, 0, 0, 1]* [oldx;oldy;oldz;1];
newx = newCoord(1);
newy = newCoord(2);
newz = newCoord(3);

end



function [extend,swLeft, swRight, newData] = inverseKinematicsSolver(A,data)
% maps a homogene transformation matrix, which describes a position and
% orientation from the fcs relative to the ccs, to the joint space
% variables
% tldr: Matrix A -> values for MCS00/01/02

% data = [sw_left;extend;sw_right;LCS;left_sph1;left_sph2;right_sph1;right_sph2]
% @fmincon
options = optimoptions(@fmincon,'OptimalityTolerance',10^-10,'StepTolerance',10^-10,'display','off','Algorithm','sqp'); % some options for more precise solution

% find out transformation matrix for way0 & LL such that the error to A is
% minimal
guessExtend = data(2); %extend

% arbitrary quess values. should be somewhat in the right area.
guessVals = [transpose(data(4:7)), guessExtend]; % [LCS,extend]

%[OptVals,fval,~,output] = fminunc(@closingWay0toTarget,guessVals,options)
clW0toTarget = @(guessVals)closingWay0toTarget(guessVals,A);
[OptVals,~,~,~] = fmincon(clW0toTarget,guessVals,[],[],[],[],[],[],@constrainsWay0,options);

% get values from way0
%OptVals = num2cell(OptVals);
%[a0,b0,c0,d0,theta0] = OptVals{:};
%again: no elegant solution in Simulink
a0 = OptVals(1);
b0 = OptVals(2);
c0 = OptVals(3);
d0 = OptVals(4);
extend = OptVals(5);

% solve closure equation with given way0

guessSwLeft = data(1); % initial guessValues for leftSw and rightSw
guessSwRight = data(3);

% guessVals = [theta1,a1_1,...,d1_2,theta2,a2_1,...,d2_2]
guessVals = [guessSwLeft, transpose(data(8:15)), guessSwRight, transpose(data(16:23))]; 
%[OptVals,fval,~,output] = fminunc(@closingEqWithGivenWay0,guessVals,options)


clEWithGivenWay0 = @(GUESSEDVALS)closingEqWithGivenWay0(GUESSEDVALS,a0,b0,c0,d0);
[OptVals,~,~,~] = fmincon(clEWithGivenWay0,guessVals,[],[],[],[],[],[],@constrainsWays1_2,options);
swLeft = OptVals(1);
swRight = OptVals(10);

newData = [swLeft;extend;swRight; a0;b0;c0;d0; transpose(OptVals(2:9)); transpose(OptVals(11:18))];
end


function [error] = closingWay0toTarget(GUESSEDVALS,MatA)
% this function returns the error between the joint values of the kinematic
% way 0 with added LegLinear ( CCS -> LCS0 --> FCS) and the joint values of
% the target matrix MatA

%GUESSEDVALS = num2cell(GUESSEDVALS);
%[a0,b0,c0,d0,theta0] = GUESSEDVALS{:};

%elegant solutions are not allowed in Simulink
a0 = GUESSEDVALS(1);
b0 = GUESSEDVALS(2);
c0 = GUESSEDVALS(3);
d0 = GUESSEDVALS(4);
theta0 = GUESSEDVALS(5);



bridgem = bridgeSubChain(a0,b0,c0,d0);
openm = openSubChain(theta0);
bridgeOpenm = bridgem * openm;
% [a,b,c,d, x,y,z] = getValuesfromHomogenMatrix(way0LLm);
% [Aa,Ab,Ac,Ad, Ax,Ay,Az] = getValuesfromHomogenMatrix(MatA);

[~,~,~,~, x,y,z] = getValuesfromHomogenMatrix(bridgeOpenm);
[~,~,~,~, Ax,Ay,Az] = getValuesfromHomogenMatrix(MatA);

error = sum(([x,y,z] - [Ax,Ay,Az]).^2); % error of the transform to A. we want to minimze this
end

function [error] = closingEqWithGivenWay0(GUESSEDVALS,a0,b0,c0,d0)
% minimize way1 and way2 for given way0 transf. --> get theta1 and theta2
%GUESSEDVALS = num2cell(GUESSEDVALS);
%[theta1,a1_1,b1_1,c1_1,d1_1, a1_2,b1_2,c1_2,d1_2, theta2,a2_1,b2_1,c2_1,d2_1, a2_2,b2_2,c2_2,d2_2] = GUESSEDVALS{:};
%again: no elegant solutions for setting variables here...
theta1 = GUESSEDVALS(1);
a1_1 = GUESSEDVALS(2);
b1_1 = GUESSEDVALS(3);
c1_1 = GUESSEDVALS(4);
d1_1 = GUESSEDVALS(5);
a1_2 = GUESSEDVALS(6);
b1_2 = GUESSEDVALS(7);
c1_2 = GUESSEDVALS(8);
d1_2 = GUESSEDVALS(9);

theta2 = GUESSEDVALS(10);
a2_1 = GUESSEDVALS(11);
b2_1 = GUESSEDVALS(12);
c2_1 = GUESSEDVALS(13);
d2_1 = GUESSEDVALS(14);
a2_2 = GUESSEDVALS(15);
b2_2 = GUESSEDVALS(16);
c2_2 = GUESSEDVALS(17);
d2_2 = GUESSEDVALS(18);


A = bridgeSubChain(a0,b0,c0,d0);
B = leftSwingSubChain(theta1, a1_1,b1_1,c1_1,d1_1, a1_2,b1_2,c1_2,d1_2);
C = rightSwingSubChain(theta2,a2_1,b2_1,c2_1,d2_1, a2_2,b2_2,c2_2,d2_2);

[Aa,Ab,Ac,Ad, Ax,Ay,Az] = getValuesfromHomogenMatrix(A);
[Ba,Bb,Bc,Bd, Bx,By,Bz] = getValuesfromHomogenMatrix(B);
[Ca,Cb,Cc,Cd, Cx,Cy,Cz] = getValuesfromHomogenMatrix(C);

Avals = [Aa,Ab,Ac,Ad, Ax,Ay,Az];
Bvals = [Ba,Bb,Bc,Bd, Bx,By,Bz];
Cvals = [Ca,Cb,Cc,Cd, Cx,Cy,Cz];

error = [Avals-Bvals, Bvals-Cvals, Cvals-Avals]; % 1x21 vector
%error = sum(error.^2) + 1000 * ((a1_1^2 + b1_1^2 + c1_1 ^2 + d1_1^2 -1)^2 + (a1_2^2 + b1_2^2 + c1_2 ^2 + d1_2^2 -1)^2 + (a2_1^2 + b2_1^2 + c2_1 ^2 + d2_1^2 -1)^2 + (a2_2^2 + b2_2^2 + c2_2^2 + d2_2^2 -1)^2 );
error = sum(error.^2);
%error = 1;
end

function [c,ceq]= constrainsWay0(x)
% make sure that solver fmincon, solves with constraint : ||quat0|| == 1
%input: x = [a0,b0,c0,d0,theta0]. with a to d parameters of quat0
c = []; % condition for ... <= 0
ceq = x(1)^2+x(2)^2+x(3)^2+x(4)^2 - 1; % condition for ||quat0|| == 1    
end

function [c,ceq]= constrainsWays1_2(x)
% make sure that solver fmincon, solves with constraint : ||quati_j|| == 1
%input: x = [theta1,a1_1,...,d1_2,theta2,a2_1,...,d2_2].
c = []; % condition for ... <= 0
ceq1_1 = x(2)^2+x(3)^2+x(4)^2+x(5)^2 - 1; % condition for ||quat1_1|| == 1
ceq1_2 = x(6)^2+x(7)^2+x(8)^2+x(9)^2 - 1; % condition for ||quat1_2|| == 1
ceq2_1 = x(11)^2+x(12)^2+x(13)^2+x(14)^2 - 1; % condition for ||quat2_1|| == 1
ceq2_2 = x(15)^2+x(16)^2+x(17)^2+x(18)^2 - 1; % condition for ||quat2_2|| == 1
ceq = [ceq1_1;ceq1_2;ceq2_1;ceq2_2];
end

function [res] = homogenTranslationMat(x,y,z)
    %this function forms a Translation about a vector x,y,z in a homogenous
    %transformation matrix res. 
    %For a Vector V: V*(x,y,z) <--> (V,1) * res
    res = [1 0 0 x; 0 1 0 y; 0 0 1 z; 0 0 0 1];
end

function [res] = quatRotM(a,b,c,d) %%Todo: use a,b,c,d as inputs
    % this function calculates the quaternion rotation matrix for given
    % vector (kx,ky,kz) and angle theta
    
    %taken from wikipedia the quaternion-derived rotation matrix, with
    %substitues a = q_r, b = q_i ...
    res = [1-2*(c^2+d^2) 2*(b*c-d*a) 2*(b*d + c*a); 2*(b*c + d*a) 1-2*(b^2+d^2) 2*(c*d - b*a); 2*(b*d-c*a) 2*(c*d+b*a) 1-2*(b^2+c^2) ];
end

function [res] = makeRotHomogen(R)
% this function fills a Rotationmatrix to make it a homogen transformation
% matrix
res = [R(1,1) R(1,2) R(1,3) 0; R(2,1) R(2,2) R(2,3) 0; R(3,1) R(3,2) R(3,3) 0; 0 0 0 1];
end

function [Weg0Mat] = bridgeSubChain(a0,b0,c0,d0)
% models the 0 subchain, includes the LCS joint
A1 = homogenTranslationMat(0.265,0,0.014);
A2 = makeRotHomogen(quatRotM(a0,b0,c0,d0));

Weg0Mat = A1 * A2;

end

function [Weg1Mat] = leftSwingSubChain(theta1, a1_1,b1_1,c1_1,d1_1, a1_2,b1_2,c1_2,d1_2)

% Transformation: HKS --> MKS01 [Chassis]
A1 = homogenTranslationMat(0.139807669447128,-0.0549998406976098,-0.051);
angle_HKS_to_MKS01 = -21.4745 * pi / 180; % the angle between the x-Axes from the HKS and the MKS01 [for theta = 0], as seen in the simulink model
                                            % todo: see if sym(pi) makes a difference
A2 = makeRotHomogen(quatRotM(cos(angle_HKS_to_MKS01/2),0,0,sin(angle_HKS_to_MKS01/2))); % quaternion matrix for rotation about z-axis

% Transformation MKS01 --> Koppelstange1_1 (1. Spherical Joint der
% Koppelstange) [Antriebshebel]
A3 = makeRotHomogen(quatRotM(cos(theta1 /2),0,0,sin(theta1 /2))); % quaternion rotation matrix for a rotation about theta [radians] about the MKS-joint-z-axis
A4 = homogenTranslationMat(0.085,0,-0.0245);

% Transformation KS1_1 --> KS1_2 (1. --> 2. Spherical Joint) [Koppelstange]
A5 = makeRotHomogen(quatRotM(a1_1, b1_1, c1_1, d1_1));
A6 = homogenTranslationMat(0.110,0,0);

% Transformation KS1_2 --> P (gemeinsamer EndPunkt aller Wege) [Antriebsmodul]
A7 = makeRotHomogen(quatRotM(a1_2, b1_2, c1_2, d1_2));
A8 = homogenTranslationMat(-0.015,0.0299,0.0965);

% Gesamt Transformationsmatrix
Weg1Mat = A1*A2*A3*A4*A5*A6*A7*A8;
end

function [Weg2Mat] = rightSwingSubChain(theta2,a2_1,b2_1,c2_1,d2_1, a2_2,b2_2,c2_2,d2_2)

% Transformation: HKS --> MKS02 [Chassis]
A1 = homogenTranslationMat(0.139807669447128,0.0549998406976098,-0.051);
angle_HKS_to_MKS02 = -338.5255 * pi / 180; % the angle between the x-Axes from the HKS and the MKS02 [for theta = 0], as seen in the simulink model
                                            % todo: see if sym(pi) makes a difference
A2 = makeRotHomogen(quatRotM(cos(angle_HKS_to_MKS02 /2),0,0,sin(angle_HKS_to_MKS02/2))); % quaternion matrix for rotation about z-axis

% Transformation MKS02 --> Koppelstange2_1 (1. Spherical Joint der
% Koppelstange) [Antriebshebel]
A3 = makeRotHomogen(quatRotM(cos(theta2 /2),0,0,sin(theta2 /2))); % quaternion rotation matrix for a rotation about theta [radians] about the MKS-joint-z-axis
A4 = homogenTranslationMat(0.085,0,-0.0245);

% Transformation KS2_1 --> KS1_2 (1. --> 2. Spherical Joint) [Koppelstange]
A5 = makeRotHomogen(quatRotM(a2_1, b2_1, c2_1, d2_1));
A6 = homogenTranslationMat(0.11,0,0);

% Transformation KS2_2 --> P (gemeinsamer EndPunkt aller Wege) [Antriebsmodul]
A7 = makeRotHomogen(quatRotM(a2_2, b2_2, c2_2, d2_2));
A8 = homogenTranslationMat(-0.015,-0.0299,0.0965);

% Gesamt Transformationsmatrix
Weg2Mat = A1*A2*A3*A4*A5*A6*A7*A8;
end

function [ALeg] = openSubChain(theta0)
% this functions adds transformations from the Point P; which is the
% Endpoint from Weg0,1,2; to the FKS at the LegLinear part.
%{
Inputs:
A: Transformationmatrix, which describes Transformation from HKS to P, Use
Weg0.m for that.
theta0: angle [radians] for joint at MKS00
%}

A_P_llJointBase = homogenTranslationMat(1.640, 0, -0.037); % transform P to P0 (center of LegLinear rotation)

startingValue = -3 * pi/180; % Zero-Offset for ExtendJoint
%theta0 = -1* theta0 * 0.07/(2*pi*1.5) + startingValue; % gear constraint: transfrom theta at MKS00 to theta around P0, gear ratio is 0.07/(2*pi*1.5) [circumferences of the gears MCS00 / LL]
theta0 = theta0 + startingValue;

A_llJoint = makeRotHomogen(quatRotM(cos(theta0/2),0,sin(theta0/2),0)); % rotate theta [radians] around y-axis of P0

A_follower_fcs = homogenTranslationMat(-1.500,0,0); %transform from P0-joint to FKS

ALeg = A_P_llJointBase*A_llJoint*A_follower_fcs;
end

function [a,b,c,d, x,y,z] = getValuesfromHomogenMatrix(A)
    % this function returns the Vector by which a homogenous
    % Transformationmatrix shifts a Point
    x = A(1,4);
    y = A(2,4);
    z = A(3,4);

    %as well as the Values of the Quaternion describing the rotation
    R = A(1:3,1:3); % get Rot mat from homogen Mat
    quati = rotm2quat(R); % convert to quaternion
    quati = quaternion(quati);
    [a,b,c,d] = parts(quati); % get parts from quaternion
    
end

== matlab function TripedLibraryMAX/Kinematic Utils/fullbody kinematics ==
  data: foot0 input; res output; foot1 input; foot2 input; roll input; pitch input; yaw input
function res  = fcn(foot0,foot1,foot2,roll,pitch,yaw)
floor_ccs_trafo=transform_to_floor(roll,pitch,yaw);

ccs_foot0=floor_ccs_trafo*makehomogenous(foot0);
ccs_foot1=floor_ccs_trafo*makehomogenous(foot1);
ccs_foot2=floor_ccs_trafo*makehomogenous(foot2);
res=[ccs_foot0(1:3);ccs_foot1(1:3);ccs_foot2(1:3)];
end


function [transfM] = transform_to_floor(roll,pitch,yaw)
%first translate frame by [x,y,z]
transfM1 = homogenTranslationMat(0,0,0);
%then rotate using the roll-pitch-yaw convention
transfM2 = makeRotHomogen(eul2rotm([-roll,-pitch,-yaw],'XYZ'));
transfM = transfM1 * transfM2;
end

function [res] = makehomogenous(vector)
    res = [vector(1);vector(2);vector(3);1];
end

function [res] = homogenTranslationMat(x,y,z)
    %this function forms a Translation about a vector x,y,z in a homogenous
    %transformation matrix res. 
    res = [1 0 0 x; 0 1 0 y; 0 0 1 z; 0 0 0 1];
end

function [res] = makeRotHomogen(R)
% this function fills a Rotationmatrix to make it a homogen transformation
% matrix
res = [R(1,1) R(1,2) R(1,3) 0; R(2,1) R(2,2) R(2,3) 0; R(3,1) R(3,2) R(3,3) 0; 0 0 0 1];
end
